simulink model "CCMSysServ"  (R2022b) (continued)
== system CCMSysServ == (continued)
      | BOSS SWITCH
      | <to>All</to>
      | <typ>Internal</typ>
      | <ReqID>NULL</ReqID>
      | <InitValue>0</InitValue>
  CarCfg_CarCfg47 : Inport  Description=|  Port=382  OutDataTypeStr=Nr2  PortDimensions=1
      | SELECTIVE CATALYTIC REDUCTION
      | <to>All</to>
      | <typ>Internal</typ>
      | <ReqID>NULL</ReqID>
      | <InitValue>0</InitValue>
  CarCfg_CarCfg470 : Inport  Description=|  Port=383  OutDataTypeStr=Nr2  PortDimensions=1
      | BELT PRE-TENSIONER 3RD ROW
      | <to>All</to>
      | <typ>Internal</typ>
      | <ReqID>NULL</ReqID>
      | <InitValue>0</InitValue>
  CarCfg_CarCfg471 : Inport  Description=|  Port=384  OutDataTypeStr=Nr2  PortDimensions=1
      | BACKREST MOTORS 1ST ROW
      | <to>All</to>
      | <typ>Internal</typ>
      | <ReqID>NULL</ReqID>
      | <InitValue>0</InitValue>
  CarCfg_CarCfg472 : Inport  Description=|  Port=385  OutDataTypeStr=Nr2  PortDimensions=1
      | FIRST ROW HEIGHT MOTORS
      | <to>All</to>
      | <typ>Internal</typ>
      | <ReqID>NULL</ReqID>
      | <InitValue>0</InitValue>
  CarCfg_CarCfg473 : Inport  Description=|  Port=386  OutDataTypeStr=Nr2  PortDimensions=1
      | SECOND ROW MASSAGE
      | <to>All</to>
      | <typ>Internal</typ>
      | <ReqID>NULL</ReqID>
      | <InitValue>0</InitValue>
  CarCfg_CarCfg474 : Inport  Description=|  Port=387  OutDataTypeStr=Nr2  PortDimensions=1
      | SECOND ROW POWER SEAT
      | <to>All</to>
      | <typ>Internal</typ>
      | <ReqID>NULL</ReqID>
      | <InitValue>0</InitValue>
  CarCfg_CarCfg475 : Inport  Description=|  Port=388  OutDataTypeStr=Nr2  PortDimensions=1
      | FIRST ROW LEG REST
      | <to>All</to>
      | <typ>Internal</typ>
      | <ReqID>NULL</ReqID>
      | <InitValue>0</InitValue>
  CarCfg_CarCfg476 : Inport  Description=|  Port=389  OutDataTypeStr=Nr2  PortDimensions=1
      | SECOND ROW LEG REST
      | <to>All</to>
      | <typ>Internal</typ>
      | <ReqID>NULL</ReqID>
      | <InitValue>0</InitValue>
  CarCfg_CarCfg477 : Inport  Description=|  Port=390  OutDataTypeStr=Nr2  PortDimensions=1
      | SECOND ROW EASY INGRESS/EGRESS
      | <to>All</to>
      | <typ>Internal</typ>
      | <ReqID>NULL</ReqID>
      | <InitValue>0</InitValue>
  CarCfg_CarCfg478 : Inport  Description=|  Port=391  OutDataTypeStr=Nr2  PortDimensions=1
      | DOOR OPENING WARNING
      | <to>All</to>
      | <typ>Internal</typ>
      | <ReqID>NULL</ReqID>
      | <InitValue>0</InitValue>
  CarCfg_CarCfg479 : Inport  Description=|  Port=392  OutDataTypeStr=Nr2  PortDimensions=1
      | FRONT CROSS TRAFFIC ALERT
      | <to>All</to>
      | <typ>Internal</typ>
      | <ReqID>NULL</ReqID>
      | <InitValue>0</InitValue>
  CarCfg_CarCfg48 : Inport  Description=|  Port=393  OutDataTypeStr=Nr2  PortDimensions=1
      | OIL LEVEL SENSOR
      | <to>All</to>
      | <typ>Internal</typ>
      | <ReqID>NULL</ReqID>
      | <InitValue>0</InitValue>
  CarCfg_CarCfg480 : Inport  Description=|  Port=394  OutDataTypeStr=Nr2  PortDimensions=1
      | NUMBER OF KEYLESS DOOR HANDLES
      | <to>All</to>
      | <typ>Internal</typ>
      | <ReqID>NULL</ReqID>
      | <InitValue>0</InitValue>
  CarCfg_CarCfg481 : Inport  Description=|  Port=395  OutDataTypeStr=Nr2  PortDimensions=1
      | POWER DOOR TYPE
      | <to>All</to>
      | <typ>Internal</typ>
      | <ReqID>NULL</ReqID>
      | <InitValue>0</InitValue>
  CarCfg_CarCfg482 : Inport  Description=|  Port=396  OutDataTypeStr=Nr2  PortDimensions=1
      | SEAT SPLIT SECOND ROW
      | <to>All</to>
      | <typ>Internal</typ>
      | <ReqID>NULL</ReqID>
      | <InitValue>0</InitValue>
  CarCfg_CarCfg483 : Inport  Description=|  Port=397  OutDataTypeStr=Nr2  PortDimensions=1
      | DIGITAL VIDEO RECORDER 
      | <to>All</to>
      | <typ>Internal</typ>
      | <ReqID>NULL</ReqID>
      | <InitValue>0</InitValue>
  CarCfg_CarCfg484 : Inport  Description=|  Port=398  OutDataTypeStr=Nr2  PortDimensions=1
      | LOGO LIGHT
      | <to>All</to>
      | <typ>Internal</typ>
      | <ReqID>NULL</ReqID>
      | <InitValue>0</InitValue>
  CarCfg_CarCfg485 : Inport  Description=|  Port=399  OutDataTypeStr=Nr2  PortDimensions=1
      | INTERIOR REAR VIEW MIRROR DISPLAY
      | <to>All</to>
      | <typ>Internal</typ>
      | <ReqID>NULL</ReqID>
      | <InitValue>0</InitValue>
  CarCfg_CarCfg486 : Inport  Description=|  Port=400  OutDataTypeStr=Nr2  PortDimensions=1
      | SECOND ROW LUMBAR AND SIDE SUPPORT
      | <to>All</to>
      | <typ>Internal</typ>
      | <ReqID>NULL</ReqID>
      | <InitValue>0</InitValue>
  CarCfg_CarCfg487 : Inport  Description=|  Port=401  OutDataTypeStr=Nr2  PortDimensions=1
      | BRAKE PEDAL ACTIVE DECOUPLING PYRO
      | <to>All</to>
      | <typ>Internal</typ>
      | <ReqID>NULL</ReqID>
      | <InitValue>0</InitValue>
  CarCfg_CarCfg488 : Inport  Description=|  Port=402  OutDataTypeStr=Nr2  PortDimensions=1
      | WIRELESS POWER TRANSFER MODULE
      | <to>All</to>
      | <typ>Internal</typ>
      | <ReqID>NULL</ReqID>
      | <InitValue>0</InitValue>
  CarCfg_CarCfg489 : Inport  Description=|  Port=403  OutDataTypeStr=Nr2  PortDimensions=1
      | BLE/NFC KEYS
      | <to>All</to>
      | <typ>Internal</typ>
      | <ReqID>NULL</ReqID>
      | <InitValue>0</InitValue>
  CarCfg_CarCfg49 : Inport  Description=|  Port=404  OutDataTypeStr=Nr2  PortDimensions=1
      | PERFORMANCE MODE
      | <to>All</to>
      | <typ>Internal</typ>
      | <ReqID>NULL</ReqID>
      | <InitValue>0</InitValue>
  CarCfg_CarCfg490 : Inport  Description=|  Port=405  OutDataTypeStr=Nr2  PortDimensions=1
      | OUTER OPENING TRIGGER OF TAILGATE
      | <to>All</to>
      | <typ>Internal</typ>
      | <ReqID>NULL</ReqID>
      | <InitValue>0</InitValue>
  CarCfg_CarCfg491 : Inport  Description=|  Port=406  OutDataTypeStr=Nr2  PortDimensions=1
      | YEAR EDITION
      | <to>All</to>
      | <typ>Internal</typ>
      | <ReqID>NULL</ReqID>
      | <InitValue>0</InitValue>
  CarCfg_CarCfg493 : Inport  Description=|  Port=407  OutDataTypeStr=Nr2  PortDimensions=1
      | EMERGENCY LANE OCCUPIED WARNING
      | <to>All</to>
      | <typ>Internal</typ>
      | <ReqID>NULL</ReqID>
      | <InitValue>0</InitValue>
  CarCfg_CarCfg494 : Inport  Description=|  Port=408  OutDataTypeStr=Nr2  PortDimensions=1
      | HIGHWAY ASSIST
      | <to>All</to>
      | <typ>Internal</typ>
      | <ReqID>NULL</ReqID>
      | <InitValue>0</InitValue>
  CarCfg_CarCfg495 : Inport  Description=|  Port=409  OutDataTypeStr=Nr2  PortDimensions=1
      | PARKING ASSISTANCE SUPPORT PEB
      | <to>All</to>
      | <typ>Internal</typ>
      | <ReqID>NULL</ReqID>
      | <InitValue>0</InitValue>
  CarCfg_CarCfg496 : Inport  Description=|  Port=410  OutDataTypeStr=Nr2  PortDimensions=1
      | BATTERY COOLANT LEVEL INDICATION
      | <to>All</to>
      | <typ>Internal</typ>
      | <ReqID>NULL</ReqID>
      | <InitValue>0</InitValue>
  CarCfg_CarCfg497 : Inport  Description=|  Port=411  OutDataTypeStr=Nr2  PortDimensions=1
      | INTERIOR VIEW CAMERA
      | <to>All</to>
      | <typ>Internal</typ>
      | <ReqID>NULL</ReqID>
      | <InitValue>0</InitValue>
  CarCfg_CarCfg498 : Inport  Description=|  Port=412  OutDataTypeStr=Nr2  PortDimensions=1
      | TEMPERATURE CONTROL CUP HOLDER
      | <to>All</to>
      | <typ>Internal</typ>
      | <ReqID>NULL</ReqID>
      | <InitValue>0</InitValue>
  CarCfg_CarCfg499 : Inport  Description=|  Port=413  OutDataTypeStr=Nr2  PortDimensions=1
      | INFOTAINMENT HEAD UNIT TYPE
      | <to>All</to>
      | <typ>Internal</typ>
      | <ReqID>NULL</ReqID>
      | <InitValue>0</InitValue>
  CarCfg_CarCfg5 : Inport  Description=|  Port=414  OutDataTypeStr=Nr2  PortDimensions=1
      | ENGINE
      | <to>All</to>
      | <typ>Internal</typ>
      | <ReqID>NULL</ReqID>
      | <InitValue>0</InitValue>
  CarCfg_CarCfg50 : Inport  Description=|  Port=415  OutDataTypeStr=Nr2  PortDimensions=1
      | CHASSIS TYPE
      | <to>All</to>
      | <typ>Internal</typ>
      | <ReqID>NULL</ReqID>
      | <InitValue>0</InitValue>
  CarCfg_CarCfg500 : Inport  Description=|  Port=416  OutDataTypeStr=Nr2  PortDimensions=1
      | DRIVER ALERT CONTROL
      | <to>All</to>
      | <typ>Internal</typ>
      | <ReqID>NULL</ReqID>
      | <InitValue>0</InitValue>
  CarCfg_CarCfg501 : Inport  Description=|  Port=417  OutDataTypeStr=Nr2  PortDimensions=1
      | WASHER FLUID LEVEL INDICATION FUNCTION
      | <to>All</to>
      | <typ>Internal</typ>
      | <ReqID>NULL</ReqID>
      | <InitValue>0</InitValue>
  CarCfg_CarCfg502 : Inport  Description=|  Port=418  OutDataTypeStr=Nr2  PortDimensions=1
      | ELECTRICAL PARK BRAKE HARD SWITCH
      | <to>All</to>
      | <typ>Internal</typ>
      | <ReqID>NULL</ReqID>
      | <InitValue>0</InitValue>
  CarCfg_CarCfg503 : Inport  Description=|  Port=419  OutDataTypeStr=Nr2  PortDimensions=1
      | WIPER MOTOR MODULE
      | <to>All</to>
      | <typ>Internal</typ>
      | <ReqID>NULL</ReqID>
      | <InitValue>0</InitValue>
  CarCfg_CarCfg504 : Inport  Description=|  Port=420  OutDataTypeStr=Nr2  PortDimensions=1
      | TRAFFIC LIGHT ATTENTION
      | <to>All</to>
      | <typ>Internal</typ>
      | <ReqID>NULL</ReqID>
      | <InitValue>0</InitValue>
  CarCfg_CarCfg505 : Inport  Description=|  Port=421  OutDataTypeStr=Nr2  PortDimensions=1
      | AIR FRAGRANCE
      | <to>All</to>
      | <typ>Internal</typ>
      | <ReqID>NULL</ReqID>
      | <InitValue>0</InitValue>
  CarCfg_CarCfg506 : Inport  Description=|  Port=422  OutDataTypeStr=Nr2  PortDimensions=1
      | ELECTRICAL VENTILATION ADJUSTMENT 
      | <to>All</to>
      | <typ>Internal</typ>
      | <ReqID>NULL</ReqID>
      | <InitValue>0</InitValue>
  CarCfg_CarCfg507 : Inport  Description=|  Port=423  OutDataTypeStr=Nr2  PortDimensions=1
      | HOOD TYPE
      | <to>All</to>
      | <typ>Internal</typ>
      | <ReqID>NULL</ReqID>
      | <InitValue>0</InitValue>
  CarCfg_CarCfg508 : Inport  Description=|  Port=424  OutDataTypeStr=Nr2  PortDimensions=1
      | REAR LAMP CONTROL MODULE
      | <to>All</to>
      | <typ>Internal</typ>
      | <ReqID>NULL</ReqID>
      | <InitValue>0</InitValue>
  CarCfg_CarCfg509 : Inport  Description=|  Port=425  OutDataTypeStr=Nr2  PortDimensions=1
      | HOME SAFE LIGHT
      | <to>All</to>
      | <typ>Internal</typ>
      | <ReqID>NULL</ReqID>
      | <InitValue>0</InitValue>
  CarCfg_CarCfg51 : Inport  Description=|  Port=426  OutDataTypeStr=Nr2  PortDimensions=1
      | TRAILER STABILITY ASSIST
      | <to>All</to>
      | <typ>Internal</typ>
      | <ReqID>NULL</ReqID>
      | <InitValue>0</InitValue>
  CarCfg_CarCfg510 : Inport  Description=|  Port=427  OutDataTypeStr=Nr2  PortDimensions=1
      | EXTERIOR LIGHT SHOW
      | <to>All</to>
      | <typ>Internal</typ>
      | <ReqID>NULL</ReqID>
      | <InitValue>0</InitValue>
  CarCfg_CarCfg511 : Inport  Description=|  Port=428  OutDataTypeStr=Nr2  PortDimensions=1
      | ADAPTIVE FRONT LIGHT SYSTEM
      | <to>All</to>
      | <typ>Internal</typ>
      | <ReqID>NULL</ReqID>
      | <InitValue>0</InitValue>
  CarCfg_CarCfg512 : Inport  Description=|  Port=429  OutDataTypeStr=Nr2  PortDimensions=1
      | SECOND BATTERY MONITORING SENSOR
      | <to>All</to>
      | <typ>Internal</typ>
      | <ReqID>NULL</ReqID>
      | <InitValue>0</InitValue>
  CarCfg_CarCfg513 : Inport  Description=|  Port=430  OutDataTypeStr=Nr2  PortDimensions=1
      | ENGINE COOLANT PUMP MODULE
      | <to>All</to>
      | <typ>Internal</typ>
      | <ReqID>NULL</ReqID>
      | <InitValue>0</InitValue>
  CarCfg_CarCfg514 : Inport  Description=|  Port=431  OutDataTypeStr=Nr2  PortDimensions=1
      | BATTERY COOLING PUMP FRONT MODULE
      | <to>All</to>
      | <typ>Internal</typ>
      | <ReqID>NULL</ReqID>
      | <InitValue>0</InitValue>
  CarCfg_CarCfg515 : Inport  Description=|  Port=432  OutDataTypeStr=Nr2  PortDimensions=1
      | HVAC BLOWER MODULE FRONT
      | <to>All</to>
      | <typ>Internal</typ>
      | <ReqID>NULL</ReqID>
      | <InitValue>0</InitValue>
  CarCfg_CarCfg516 : Inport  Description=|  Port=433  OutDataTypeStr=Nr2  PortDimensions=1
      | DRIVETRAIN COOLANT PUMP
      | <to>All</to>
      | <typ>Internal</typ>
      | <ReqID>NULL</ReqID>
      | <InitValue>0</InitValue>
  CarCfg_CarCfg517 : Inport  Description=|  Port=434  OutDataTypeStr=Nr2  PortDimensions=1
      | ELECTRIC DRIVETRAIN COOLANT PUMP
      | <to>All</to>
      | <typ>Internal</typ>
      | <ReqID>NULL</ReqID>
      | <InitValue>0</InitValue>
  CarCfg_CarCfg518 : Inport  Description=|  Port=435  OutDataTypeStr=Nr2  PortDimensions=1
      | OCCUPANT DETECTION SWITCHES 3RD ROW
      | <to>All</to>
      | <typ>Internal</typ>
      | <ReqID>NULL</ReqID>
      | <InitValue>0</InitValue>
  CarCfg_CarCfg519 : Inport  Description=|  Port=436  OutDataTypeStr=Nr2  PortDimensions=1
      | VEHICLE PERSONA THEME
      | <to>All</to>
      | <typ>Internal</typ>
      | <ReqID>NULL</ReqID>
      | <InitValue>0</InitValue>
  CarCfg_CarCfg52 : Inport  Description=|  Port=437  OutDataTypeStr=Nr2  PortDimensions=1
      | POST-IMPACT BRAKING
      | <to>All</to>
      | <typ>Internal</typ>
      | <ReqID>NULL</ReqID>
      | <InitValue>0</InitValue>
  CarCfg_CarCfg520 : Inport  Description=|  Port=438  OutDataTypeStr=Nr2  PortDimensions=1
      | 2ND ROW SIDE AIRBAGS
      | <to>All</to>
      | <typ>Internal</typ>
      | <ReqID>NULL</ReqID>
      | <InitValue>0</InitValue>
  CarCfg_CarCfg521 : Inport  Description=|  Port=439  OutDataTypeStr=Nr2  PortDimensions=1
      | SEAT MATERIAL FOR AUDIO TUNING
      | <to>All</to>
      | <typ>Internal</typ>
      | <ReqID>NULL</ReqID>
      | <InitValue>0</InitValue>
  CarCfg_CarCfg522 : Inport  Description=|  Port=440  OutDataTypeStr=Nr2  PortDimensions=1
      | 3RD ROW SEAT MATERIAL FOR AUDIO TUNING
      | <to>All</to>
      | <typ>Internal</typ>
      | <ReqID>NULL</ReqID>
      | <InitValue>0</InitValue>
  CarCfg_CarCfg523 : Inport  Description=|  Port=441  OutDataTypeStr=Nr2  PortDimensions=1
      | DRIVER DOOR SWITCH TYPE
      | <to>All</to>
      | <typ>Internal</typ>
      | <ReqID>NULL</ReqID>
      | <InitValue>0</InitValue>
  CarCfg_CarCfg524 : Inport  Description=|  Port=442  OutDataTypeStr=Nr2  PortDimensions=1
      | TRANSMISSION LOCKING
      | <to>All</to>
      | <typ>Internal</typ>
      | <ReqID>NULL</ReqID>
      | <InitValue>0</InitValue>
  CarCfg_CarCfg525 : Inport  Description=|  Port=443  OutDataTypeStr=Nr2  PortDimensions=1
      | MOOD LIGHT
      | <to>All</to>
      | <typ>Internal</typ>
      | <ReqID>NULL</ReqID>
      | <InitValue>0</InitValue>
  CarCfg_CarCfg526 : Inport  Description=|  Port=444  OutDataTypeStr=Nr2  PortDimensions=1
      | READING LIGHT
      | <to>All</to>
      | <typ>Internal</typ>
      | <ReqID>NULL</ReqID>
      | <InitValue>0</InitValue>
  CarCfg_CarCfg527 : Inport  Description=|  Port=445  OutDataTypeStr=Nr2  PortDimensions=1
      | VEHICLE TYPE
      | <to>All</to>
      | <typ>Internal</typ>
      | <ReqID>NULL</ReqID>
      | <InitValue>0</InitValue>
  CarCfg_CarCfg528 : Inport  Description=|  Port=446  OutDataTypeStr=Nr2  PortDimensions=1
      | VEHICLE TYPE
      | <to>All</to>
      | <typ>Internal</typ>
      | <ReqID>NULL</ReqID>
      | <InitValue>0</InitValue>
  CarCfg_CarCfg529 : Inport  Description=|  Port=447  OutDataTypeStr=Nr2  PortDimensions=1
      | VEHICLE TYPE
      | <to>All</to>
      | <typ>Internal</typ>
      | <ReqID>NULL</ReqID>
      | <InitValue>0</InitValue>
  CarCfg_CarCfg53 : Inport  Description=|  Port=448  OutDataTypeStr=Nr2  PortDimensions=1
      | HILL DESCENT CONTROL
      | <to>All</to>
      | <typ>Internal</typ>
      | <ReqID>NULL</ReqID>
      | <InitValue>0</InitValue>
  CarCfg_CarCfg531 : Inport  Description=|  Port=449  OutDataTypeStr=Nr2  PortDimensions=1
      | DRIVER DOOR AND LATCH TYPE
      | <to>All</to>
      | <typ>Internal</typ>
      | <ReqID>NULL</ReqID>
      | <InitValue>0</InitValue>
  CarCfg_CarCfg532 : Inport  Description=|  Port=450  OutDataTypeStr=Nr2  PortDimensions=1
      | PASSENGER DOOR AND LATCH TYPE
      | <to>All</to>
      | <typ>Internal</typ>
      | <ReqID>NULL</ReqID>
      | <InitValue>0</InitValue>
  CarCfg_CarCfg533 : Inport  Description=|  Port=451  OutDataTypeStr=Nr2  PortDimensions=1
      | REAR LEFT DOOR AND LATCH TYPE
      | <to>All</to>
      | <typ>Internal</typ>
      | <ReqID>NULL</ReqID>
      | <InitValue>0</InitValue>
  CarCfg_CarCfg534 : Inport  Description=|  Port=452  OutDataTypeStr=Nr2  PortDimensions=1
      | REAR RIGHT DOOR AND LATCH TYPE
      | <to>All</to>
      | <typ>Internal</typ>
      | <ReqID>NULL</ReqID>
      | <InitValue>0</InitValue>
  CarCfg_CarCfg535 : Inport  Description=|  Port=453  OutDataTypeStr=Nr2  PortDimensions=1
      | ELECTRICAL PARKING BRAKE CONTROL
      | <to>All</to>
      | <typ>Internal</typ>
      | <ReqID>947612</ReqID>
      | <InitValue>0</InitValue>
  CarCfg_CarCfg536 : Inport  Description=|  Port=454  OutDataTypeStr=Nr2  PortDimensions=1
      | AUTOSTART 2ND ROW CLIMATE
      | <to>All</to>
      | <typ>Internal</typ>
      | <ReqID>NULL</ReqID>
      | <InitValue>0</InitValue>
  CarCfg_CarCfg54 : Inport  Description=|  Port=455  OutDataTypeStr=Nr2  PortDimensions=1
      | WHEEL BRAKES TYPE REAR
      | <to>All</to>
      | <typ>Internal</typ>
      | <ReqID>NULL</ReqID>
      | <InitValue>0</InitValue>
  CarCfg_CarCfg540 : Inport  Port=456  OutDataTypeStr=Nr2  PortDimensions=1
  CarCfg_CarCfg541 : Inport  Description=|  Port=457  OutDataTypeStr=Nr2  PortDimensions=1
      | POW STEERING WHEEL POSITION ADJUSTMENT
      | <to>All</to>
      | <typ>Internal</typ>
      | <ReqID>NULL</ReqID>
      | <InitValue>0</InitValue>
  CarCfg_CarCfg543 : Inport  Description=|  Port=458  OutDataTypeStr=Nr2  PortDimensions=1
      | BATTERY BACKED-UP SOUNDER TYPE
      | <to>All</to>
      | <typ>Internal</typ>
      | <ReqID>NULL</ReqID>
      | <InitValue>0</InitValue>
  CarCfg_CarCfg544 : Inport  Description=|  Port=459  OutDataTypeStr=Nr2  PortDimensions=1
      | EXTERIOR REAR VIEW MIRROR DISPLAY
      | <to>All</to>
      | <typ>Internal</typ>
      | <ReqID>NULL</ReqID>
      | <InitValue>0</InitValue>
  CarCfg_CarCfg545 : Inport  Description=|  Port=460  OutDataTypeStr=Nr2  PortDimensions=1
      | BRAKE PEDAL SENSOR/SWITCH
      | <to>All</to>
      | <typ>Internal</typ>
      | <ReqID>NULL</ReqID>
      | <InitValue>0</InitValue>
  CarCfg_CarCfg546 : Inport  Description=|  Port=461  OutDataTypeStr=Nr2  PortDimensions=1
      | ILLUMINATION SWITCH TYPE
      | <to>All</to>
      | <typ>Internal</typ>
      | <ReqID>NULL</ReqID>
      | <InitValue>0</InitValue>
  CarCfg_CarCfg547 : Inport  Port=462  OutDataTypeStr=Nr2  PortDimensions=1
  CarCfg_CarCfg55 : Inport  Description=|  Port=463  OutDataTypeStr=Nr2  PortDimensions=1
      | WHEEL BRAKES TYPE FRONT
      | <to>All</to>
      | <typ>Internal</typ>
      | <ReqID>NULL</ReqID>
      | <InitValue>0</InitValue>
  CarCfg_CarCfg550 : Inport  Description=|  Port=464  OutDataTypeStr=Nr2  PortDimensions=1
      | LUMBAR CONTROL
      | <to>All</to>
      | <typ>Internal</typ>
      | <ReqID>667104</ReqID>
      | <InitValue>0</InitValue>
  CarCfg_CarCfg551 : Inport  Description=|  Port=465  OutDataTypeStr=Nr2  PortDimensions=1
      | SECOND ROW MEMORY SEAT
      | <to>All</to>
      | <typ>Internal</typ>
      | <ReqID>NULL</ReqID>
      | <InitValue>0</InitValue>
  CarCfg_CarCfg56 : Inport  Description=|  Port=466  OutDataTypeStr=Nr2  PortDimensions=1
      | BRAKE PADS
      | <to>All</to>
      | <typ>Internal</typ>
      | <ReqID>NULL</ReqID>
      | <InitValue>0</InitValue>
  CarCfg_CarCfg561 : Inport  Description=|  Port=467  OutDataTypeStr=Nr2  PortDimensions=1
      | DOOR WINDOWS TYPE
      | <to>All</to>
      | <typ>Internal</typ>
      | <ReqID>NULL</ReqID>
      | <InitValue>0</InitValue>
  CarCfg_CarCfg564 : Inport  Description=|  Port=468  OutDataTypeStr=Nr2  PortDimensions=1
      | ACTIVE REAR SPOILER
      | <to>All</to>
      | <typ>Internal</typ>
      | <ReqID>NULL</ReqID>
      | <InitValue>0</InitValue>
  CarCfg_CarCfg565 : Inport  Description=|  Port=469  OutDataTypeStr=Nr2  PortDimensions=1
      | REMOTE STRAIGHT PARKING ASSIST
      | <to>All</to>
      | <typ>Internal</typ>
      | <ReqID>NULL</ReqID>
      | <InitValue>0</InitValue>
  CarCfg_CarCfg566 : Inport  Description=|  Port=470  OutDataTypeStr=Nr2  PortDimensions=1
      | VEHICLE TYPE
      | <to>All</to>
      | <typ>Internal</typ>
      | <ReqID>NULL</ReqID>
      | <InitValue>0</InitValue>
  CarCfg_CarCfg567 : Inport  Description=|  Port=471  OutDataTypeStr=Nr2  PortDimensions=1
      | ELECTRIC VEHICLE INLET TYPE
      | <to>All</to>
      | <typ>Internal</typ>
      | <ReqID>NULL</ReqID>
      | <InitValue>0</InitValue>
  CarCfg_CarCfg568 : Inport  Description=|  Port=472  OutDataTypeStr=Nr2  PortDimensions=1
      | LIFE DETECTION AND CARE
      | <to>All</to>
      | <typ>Internal</typ>
      | <ReqID>NULL</ReqID>
      | <InitValue>0</InitValue>
  CarCfg_CarCfg569 : Inport  Description=|  Port=473  OutDataTypeStr=Nr2  PortDimensions=1
      | REDUNDANT LV ENERGY SOURCE
      | <to>All</to>
      | <typ>Internal</typ>
      | <ReqID>21966</ReqID>
      | <InitValue>0</InitValue>
  CarCfg_CarCfg57 : Inport  Description=|  Port=474  OutDataTypeStr=Nr2  PortDimensions=1
      | DRIVE MODE
      | <to>All</to>
      | <typ>Internal</typ>
      | <ReqID>NULL</ReqID>
      | <InitValue>0</InitValue>
  CarCfg_CarCfg578 : Inport  Description=|  Port=475  OutDataTypeStr=Nr2  PortDimensions=1
      | DC CHARGE LID LOCK
      | <to>All</to>
      | <typ>Internal</typ>
      | <ReqID>NULL</ReqID>
      | <InitValue>0</InitValue>
  CarCfg_CarCfg579 : Inport  Description=|  Port=476  OutDataTypeStr=Nr2  PortDimensions=1
      | VEHICLE TYPE
      | <to>All</to>
      | <typ>Internal</typ>
      | <ReqID>NULL</ReqID>
      | <InitValue>0</InitValue>
  CarCfg_CarCfg58 : Inport  Description=|  Port=477  OutDataTypeStr=Nr2  PortDimensions=1
      | AIR SUSPENSION
      | <to>All</to>
      | <typ>Internal</typ>
      | <ReqID>NULL</ReqID>
      | <InitValue>0</InitValue>
  CarCfg_CarCfg580 : Inport  Description=|  Port=478  OutDataTypeStr=Nr2  PortDimensions=1
      | VEHICLE TYPE
      | <to>All</to>
      | <typ>Internal</typ>
      | <ReqID>NULL</ReqID>
      | <InitValue>0</InitValue>
  CarCfg_CarCfg59 : Inport  Description=|  Port=479  OutDataTypeStr=Nr2  PortDimensions=1
      | ACTIVE SUSPENSION
      | <to>All</to>
      | <typ>Internal</typ>
      | <ReqID>NULL</ReqID>
      | <InitValue>0</InitValue>
  CarCfg_CarCfg590 : Inport  Description=|  Port=480  OutDataTypeStr=Nr2  PortDimensions=1
      | MAIN FUEL OR CHARGE LID LOCATION
      | <to>All</to>
      | <typ>Internal</typ>
      | <ReqID>56442</ReqID>
      | <InitValue>0</InitValue>
  CarCfg_CarCfg591 : Inport  Description=|  Port=481  OutDataTypeStr=Nr2  PortDimensions=1
      | SECOND CHARGE LID LOCATION
      | <to>All</to>
      | <typ>Internal</typ>
      | <ReqID>56442</ReqID>
      | <InitValue>0</InitValue>
  CarCfg_CarCfg593 : Inport  Description=|  Port=482  OutDataTypeStr=Nr2  PortDimensions=1
      | SUPPORT LV ENERGY SOURCE
      | <to>All</to>
      | <typ>Internal</typ>
      | <ReqID>NULL</ReqID>
      | <InitValue>0</InitValue>
  CarCfg_CarCfg595 : Inport  Description=|  Port=483  OutDataTypeStr=Nr2  PortDimensions=1
      | Architecture type of all-electric charging cover
      | <to>All</to>
      | <typ>Internal</typ>
      | <ReqID>NULL</ReqID>
      | <InitValue>0</InitValue>
  CarCfg_CarCfg599 : Inport  Description=|  Port=484  OutDataTypeStr=Nr2  PortDimensions=1
      | VEHICLE TYPE
      | <to>All</to>
      | <typ>Internal</typ>
      | <ReqID>NULL</ReqID>
      | <InitValue>0</InitValue>
  CarCfg_CarCfg6 : Inport  Description=|  Port=485  OutDataTypeStr=Nr2  PortDimensions=1
      | FUEL
      | <to>All</to>
      | <typ>Internal</typ>
      | <ReqID>NULL</ReqID>
      | <InitValue>0</InitValue>
  CarCfg_CarCfg60 : Inport  Description=|  Port=486  OutDataTypeStr=Nr2  PortDimensions=1
      | LEVELLING SENSOR CONFIGURATION
      | <to>All</to>
      | <typ>Internal</typ>
      | <ReqID>NULL</ReqID>
      | <InitValue>0</InitValue>
  CarCfg_CarCfg604 : Inport  Description=|  Port=487  OutDataTypeStr=Nr2  PortDimensions=1
      | VEHICLE TYPE
      | <to>All</to>
      | <typ>Internal</typ>
      | <ReqID>NULL</ReqID>
      | <InitValue>0</InitValue>
  CarCfg_CarCfg609 : Inport  Description=|  Port=488  OutDataTypeStr=Nr2  PortDimensions=1
      | VEHICLE TYPE
      | <to>All</to>
      | <typ>Internal</typ>
      | <ReqID>NULL</ReqID>
      | <InitValue>0</InitValue>
  CarCfg_CarCfg61 : Inport  Description=|  Port=489  OutDataTypeStr=Nr2  PortDimensions=1
      | HILL START ASSIST
      | <to>All</to>
      | <typ>Internal</typ>
      | <ReqID>NULL</ReqID>
      | <InitValue>0</InitValue>
  CarCfg_CarCfg611 : Inport  Description=|  Port=490  OutDataTypeStr=Nr2  PortDimensions=1
      | Refresh mode
      | <to>All</to>
      | <typ>Internal</typ>
      | <ReqID>NULL</ReqID>
      | <InitValue>0</InitValue>
  CarCfg_CarCfg613 : Inport  Description=|  Port=491  OutDataTypeStr=Nr2  PortDimensions=1
      | Romantic Mode
      | <to>All</to>
      | <typ>Internal</typ>
      | <ReqID>NULL</ReqID>
      | <InitValue>0</InitValue>
  CarCfg_CarCfg615 : Inport  Description=|  Port=492  OutDataTypeStr=Nr2  PortDimensions=1
      | ECO+ mode
      | <to>All</to>
      | <typ>Internal</typ>
      | <ReqID>NULL</ReqID>
      | <InitValue>0</InitValue>
  CarCfg_CarCfg616 : Inport  Description=|  Port=493  OutDataTypeStr=Nr2  PortDimensions=1
      | Rest Mode
      | <to>All</to>
      | <typ>Internal</typ>
      | <ReqID>NULL</ReqID>
      | <InitValue>0</InitValue>
  CarCfg_CarCfg617 : Inport  Description=|  Port=494  OutDataTypeStr=Nr2  PortDimensions=1
      | Theater mode
      | <to>All</to>
      | <typ>Internal</typ>
      | <ReqID>NULL</ReqID>
      | <InitValue>0</InitValue>
  CarCfg_CarCfg618 : Inport  Description=|  Port=495  OutDataTypeStr=Nr2  PortDimensions=1
      | Vehicle Sentry and Theft Deterrence
      | <to>UsgModMgr</to>
      | <typ>Internal</typ>
      | <ReqID>22019</ReqID>
      | <InitValue>0</InitValue>
  CarCfg_CarCfg62 : Inport  Description=|  Port=496  OutDataTypeStr=Nr2  PortDimensions=1
      | FRONT AXLE WEIGHT
      | <to>All</to>
      | <typ>Internal</typ>
      | <ReqID>NULL</ReqID>
      | <InitValue>0</InitValue>
  CarCfg_CarCfg622 : Inport  Description=|  Port=497  OutDataTypeStr=Nr2  PortDimensions=1
      | Grille Lamp
      | <to>All</to>
      | <typ>Internal</typ>
      | <ReqID>682377</ReqID>
      | <InitValue>0</InitValue>
  CarCfg_CarCfg624 : Inport  Description=|  Port=498  OutDataTypeStr=Nr2  PortDimensions=1
      | RPA TYPE
      | <to>All</to>
      | <typ>Internal</typ>
      | <ReqID>NULL</ReqID>
      | <InitValue>0</InitValue>
  CarCfg_CarCfg626 : Inport  Description=|  Port=499  OutDataTypeStr=Nr2  PortDimensions=1
      | Customization mode
      | <to>All</to>
      | <typ>Internal</typ>
      | <ReqID>NULL</ReqID>
      | <InitValue>0</InitValue>
  CarCfg_CarCfg627 : Inport  Description=|  Port=500  OutDataTypeStr=Nr2  PortDimensions=1
      | Meeting Mode
      | <to>All</to>
      | <typ>Internal</typ>
      | <ReqID>NULL</ReqID>
      | <InitValue>0</InitValue>
  CarCfg_CarCfg628 : Inport  Description=|  Port=501  OutDataTypeStr=Nr2  PortDimensions=1
      | Digital Key Protocol
      | <to>All</to>
      | <typ>Internal</typ>
      | <ReqID>NULL</ReqID>
      | <InitValue>0</InitValue>
  CarCfg_CarCfg629 : Inport  Description=|  Port=502  OutDataTypeStr=Nr2  PortDimensions=1
      | EXTERIOR Light Switch Type
      | <to>All</to>
      | <typ>Internal</typ>
      | <ReqID>21928</ReqID>
      | <InitValue>0</InitValue>
  CarCfg_CarCfg63 : Inport  Description=|  Port=503  OutDataTypeStr=Nr2  PortDimensions=1
      | REAR AXLE WEIGHT
      | <to>All</to>
      | <typ>Internal</typ>
      | <ReqID>NULL</ReqID>
      | <InitValue>0</InitValue>
  CarCfg_CarCfg630 : Inport  Description=|  Port=504  OutDataTypeStr=Nr2  PortDimensions=1
      | Remote Pilot Function
      | <to>All</to>
      | <typ>Internal</typ>
      | <ReqID>267298</ReqID>
      | <InitValue>0</InitValue>
  CarCfg_CarCfg634 : Inport  Description=|  Port=505  OutDataTypeStr=Nr2  PortDimensions=1
      | RPA TYPE
      | <to>All</to>
      | <typ>Internal</typ>
      | <ReqID>NULL</ReqID>
      | <InitValue>0</InitValue>
  CarCfg_CarCfg635 : Inport  Description=|  Port=506  OutDataTypeStr=Nr2  PortDimensions=1
      | Architecture type of all-electric charging cover
      | <to>All</to>
      | <typ>Internal</typ>
      | <ReqID>NULL</ReqID>
      | <InitValue>0</InitValue>
  CarCfg_CarCfg639 : Inport  Description=|  Port=507  OutDataTypeStr=Nr2  PortDimensions=1
      | AVP TYPE
      | <to>All</to>
      | <typ>Internal</typ>
      | <ReqID>NULL</ReqID>
      | <InitValue>0</InitValue>
  CarCfg_CarCfg64 : Inport  Description=|  Port=508  OutDataTypeStr=Nr2  PortDimensions=1
      | ALARM
      | <to>All</to>
      | <typ>Internal</typ>
      | <ReqID>NULL</ReqID>
      | <InitValue>0</InitValue>
  CarCfg_CarCfg640 : Inport  Description=|  Port=509  OutDataTypeStr=Nr2  PortDimensions=1
      | RPA TYPE
      | <to>All</to>
      | <typ>Internal</typ>
      | <ReqID>NULL</ReqID>
      | <InitValue>0</InitValue>
  CarCfg_CarCfg642 : Inport  Description=|  Port=510  OutDataTypeStr=Nr2  PortDimensions=1
      | Active rear spoiler type
      | <to>All</to>
      | <typ>Internal</typ>
      | <ReqID>512424</ReqID>
      | <InitValue>0</InitValue>
  CarCfg_CarCfg644 : Inport  Description=|  Port=511  OutDataTypeStr=Nr2  PortDimensions=1
      | Architecture type of all-electric charging cover
      | <to>All</to>
      | <typ>Internal</typ>
      | <ReqID>NULL</ReqID>
      | <InitValue>0</InitValue>
  CarCfg_CarCfg645 : Inport  Description=|  Port=512  OutDataTypeStr=Nr2  PortDimensions=1
      | Architecture type of all-electric charging cover
      | <to>All</to>
      | <typ>Internal</typ>
      | <ReqID>NULL</ReqID>
      | <InitValue>0</InitValue>
  CarCfg_CarCfg646 : Inport  Description=|  Port=513  OutDataTypeStr=Nr2  PortDimensions=1
      | Architecture type of all-electric charging cover
      | <to>All</to>
      | <typ>Internal</typ>
      | <ReqID>NULL</ReqID>
      | <InitValue>0</InitValue>
  CarCfg_CarCfg647 : Inport  Description=|  Port=514  OutDataTypeStr=Nr2  PortDimensions=1
      | Side step light
      | <to>All</to>
      | <typ>Internal</typ>
      | <ReqID>56442</ReqID>
      | <InitValue>0</InitValue>
  CarCfg_CarCfg648 : Inport  Description=|  Port=515  OutDataTypeStr=Nr2  PortDimensions=1
      | VEHICLE TYPE
      | <to>All</to>
      | <typ>Internal</typ>
      | <ReqID>NULL</ReqID>
      | <InitValue>0</InitValue>
  CarCfg_CarCfg65 : Inport  Description=|  Port=516  OutDataTypeStr=Nr2  PortDimensions=1
      | INTERIOR MOTION SENSOR
      | <to>All</to>
      | <typ>Internal</typ>
      | <ReqID>NULL</ReqID>
      | <InitValue>0</InitValue>
  CarCfg_CarCfg650 : Inport  Description=|  Port=517  OutDataTypeStr=Nr2  PortDimensions=1
      | EXTERNAL VOICE INTERACTION
      | <to>All</to>
      | <typ>Internal</typ>
      | <ReqID>NULL</ReqID>
      | <InitValue>0</InitValue>
  CarCfg_CarCfg651 : Inport  Description=|  Port=518  OutDataTypeStr=Nr2  PortDimensions=1
      | ELECTRIC VEHICLE INLET TYPE
      | <to>All</to>
      | <typ>Internal</typ>
      | <ReqID>NULL</ReqID>
      | <InitValue>0</InitValue>
  CarCfg_CarCfg652 : Inport  Description=|  Port=519  OutDataTypeStr=Nr2  PortDimensions=1
      | Rear door handle switch type
      | <to>All</to>
      | <typ>Internal</typ>
      | <ReqID>NULL</ReqID>
      | <InitValue>0</InitValue>
  CarCfg_CarCfg659 : Inport  Description=|  Port=520  OutDataTypeStr=Nr2  PortDimensions=1
      | VEHICLE TYPE
      | <to>All</to>
      | <typ>Internal</typ>
      | <ReqID>NULL</ReqID>
      | <InitValue>0</InitValue>
  CarCfg_CarCfg66 : Inport  Description=|  Port=521  OutDataTypeStr=Nr2  PortDimensions=1
      | INCLINATION SENSOR
      | <to>All</to>
      | <typ>Internal</typ>
      | <ReqID>NULL</ReqID>
      | <InitValue>0</InitValue>
  CarCfg_CarCfg660 : Inport  Description=|  Port=522  OutDataTypeStr=Nr2  PortDimensions=1
      | VEHICLE TYPE
      | <to>All</to>
      | <typ>Internal</typ>
      | <ReqID>NULL</ReqID>
      | <InitValue>0</InitValue>
  CarCfg_CarCfg665 : Inport  Description=|  Port=523  OutDataTypeStr=Nr2  PortDimensions=1
      | VEHICLE TYPE
      | <to>All</to>
      | <typ>Internal</typ>
      | <ReqID>NULL</ReqID>
      | <InitValue>0</InitValue>
  CarCfg_CarCfg67 : Inport  Description=|  Port=524  OutDataTypeStr=Nr2  PortDimensions=1
      | STEERING COLUMN LOCK
      | <to>All</to>
      | <typ>Internal</typ>
      | <ReqID>NULL</ReqID>
      | <InitValue>0</InitValue>
  CarCfg_CarCfg671 : Inport  Description=|  Port=525  OutDataTypeStr=Nr2  PortDimensions=1
      | Generation of power side door
      | <to>All</to>
      | <typ>Internal</typ>
      | <ReqID>301295</ReqID>
      | <InitValue>0</InitValue>
  CarCfg_CarCfg672 : Inport  Description=|  Port=526  OutDataTypeStr=Nr2  PortDimensions=1
      | Gesture Interaction
      | <to>All</to>
      | <typ>Internal</typ>
      | <ReqID>526878</ReqID>
      | <InitValue>0</InitValue>
  CarCfg_CarCfg675 : Inport  Description=|  Port=527  OutDataTypeStr=Nr2  PortDimensions=1
      | ELECTRIC RETRACTABLE STEP
      | <to>All</to>
      | <typ>Internal</typ>
      | <ReqID>56442</ReqID>
      | <InitValue>0</InitValue>
  CarCfg_CarCfg677 : Inport  Port=528  OutDataTypeStr=Nr2  PortDimensions=1
  CarCfg_CarCfg678 : Inport  Port=529  OutDataTypeStr=Nr2  PortDimensions=1
  CarCfg_CarCfg68 : Inport  Description=|  Port=530  OutDataTypeStr=Nr2  PortDimensions=1
      | PANIC FUNCTION
      | <to>All</to>
      | <typ>Internal</typ>
      | <ReqID>NULL</ReqID>
      | <InitValue>0</InitValue>
  CarCfg_CarCfg683 : Inport  Description=|  Port=531  OutDataTypeStr=Nr2  PortDimensions=1
      | /
      | <to>All</to>
      | <typ>Internal</typ>
      | <ReqID>NULL</ReqID>
      | <InitValue>0</InitValue>
  CarCfg_CarCfg684 : Inport  Description=|  Port=532  OutDataTypeStr=Nr2  PortDimensions=1
      | VEHICLE TYPE
      | <to>All</to>
      | <typ>Internal</typ>
      | <ReqID>NULL</ReqID>
      | <InitValue>0</InitValue>
  CarCfg_CarCfg686 : Inport  Description=|  Port=533  OutDataTypeStr=Nr2  PortDimensions=1
      | Architecture type of all-electric charging cover
      | <to>All</to>
      | <typ>Internal</typ>
      | <ReqID>NULL</ReqID>
      | <InitValue>0</InitValue>
  CarCfg_CarCfg687 : Inport  Description=|  Port=534  OutDataTypeStr=Nr2  PortDimensions=1
      | Architecture type of all-electric charging cover
      | <to>All</to>
      | <typ>Internal</typ>
      | <ReqID>NULL</ReqID>
      | <InitValue>0</InitValue>
  CarCfg_CarCfg688 : Inport  Description=|  Port=535  OutDataTypeStr=Nr2  PortDimensions=1
      | VEHICLE TYPE
      | <to>All</to>
      | <typ>Internal</typ>
      | <ReqID>NULL</ReqID>
      | <InitValue>0</InitValue>
  CarCfg_CarCfg69 : Inport  Description=|  Port=536  OutDataTypeStr=Nr2  PortDimensions=1
      | RETRIGG ENABLING, IF ALARM
      | <to>All</to>
      | <typ>Internal</typ>
      | <ReqID>NULL</ReqID>
      | <InitValue>0</InitValue>
  CarCfg_CarCfg695 : Inport  Description=|  Port=537  OutDataTypeStr=Nr2  PortDimensions=1
      | VEHICLE TYPE
      | <to>All</to>
      | <typ>Internal</typ>
      | <ReqID>NULL</ReqID>
      | <InitValue>0</InitValue>
  CarCfg_CarCfg697 : Inport  Description=|  Port=538  OutDataTypeStr=Nr2  PortDimensions=1
      | VEHICLE TYPE
      | <to>All</to>
      | <typ>Internal</typ>
      | <ReqID>NULL</ReqID>
      | <InitValue>0</InitValue>
  CarCfg_CarCfg698 : Inport  Description=|  Port=539  OutDataTypeStr=Nr2  PortDimensions=1
      | Architecture type of all-electric charging cover
      | <to>All</to>
      | <typ>Internal</typ>
      | <ReqID>NULL</ReqID>
      | <InitValue>0</InitValue>
  CarCfg_CarCfg699 : Inport  Description=|  Port=540  OutDataTypeStr=Nr2  PortDimensions=1
      | Wading Assist System
      | <to>All</to>
      | <typ>Internal</typ>
      | <ReqID>710182</ReqID>
      | <InitValue>0</InitValue>
  CarCfg_CarCfg70 : Inport  Description=|  Port=541  OutDataTypeStr=Nr2  PortDimensions=1
      | PASSIVE ARMING
      | <to>All</to>
      | <typ>Internal</typ>
      | <ReqID>NULL</ReqID>
      | <InitValue>0</InitValue>
  CarCfg_CarCfg701 : Inport  Description=|  Port=542  OutDataTypeStr=Nr2  PortDimensions=1
      | VEHICLE TYPE
      | <to>All</to>
      | <typ>Internal</typ>
      | <ReqID>NULL</ReqID>
      | <InitValue>0</InitValue>
  CarCfg_CarCfg705 : Inport  Description=|  Port=543  OutDataTypeStr=Nr2  PortDimensions=1
      | Architecture type of all-electric charging cover
      | <to>All</to>
      | <typ>Internal</typ>
      | <ReqID>NULL</ReqID>
      | <InitValue>0</InitValue>
  CarCfg_CarCfg709 : Inport  Description=|  Port=544  OutDataTypeStr=Nr2  PortDimensions=1
      | BRAND
      | <to>All</to>
      | <typ>Internal</typ>
      | <ReqID>700858</ReqID>
      | <InitValue>0</InitValue>
  CarCfg_CarCfg71 : Inport  Description=|  Port=545  OutDataTypeStr=Nr2  PortDimensions=1
      | FOREIGN COMPONENT DETECTION
      | <to>All</to>
      | <typ>Internal</typ>
      | <ReqID>NULL</ReqID>
      | <InitValue>0</InitValue>
  CarCfg_CarCfg714 : Inport  Description=|  Port=546  OutDataTypeStr=Nr2  PortDimensions=1
      | Rear Windshild electric Heating Defrosting
      | <to>All</to>
      | <typ>Internal</typ>
      | <ReqID>86032</ReqID>
      | <InitValue>0</InitValue>
  CarCfg_CarCfg715 : Inport  Description=|  Port=547  OutDataTypeStr=Nr2  PortDimensions=1
      | Lighting scene mode
      | <to>All</to>
      | <typ>Internal</typ>
      | <ReqID>699482</ReqID>
      | <InitValue>0</InitValue>
  CarCfg_CarCfg718 : Inport  Description=|  Port=548  OutDataTypeStr=Nr2  PortDimensions=1
      | /
      | <to>All</to>
      | <typ>Internal</typ>
      | <ReqID>NULL</ReqID>
      | <InitValue>0</InitValue>
  CarCfg_CarCfg719 : Inport  Description=|  Port=549  OutDataTypeStr=Nr2  PortDimensions=1
      | Architecture type of all-electric charging cover
      | <to>All</to>
      | <typ>Internal</typ>
      | <ReqID>NULL</ReqID>
      | <InitValue>0</InitValue>
  CarCfg_CarCfg72 : Inport  Description=|  Port=550  OutDataTypeStr=Nr2  PortDimensions=1
      | IMMOBILISER VARIANT
      | <to>All</to>
      | <typ>Internal</typ>
      | <ReqID>NULL</ReqID>
      | <InitValue>0</InitValue>
  CarCfg_CarCfg720 : Inport  Description=|  Port=551  OutDataTypeStr=Nr2  PortDimensions=1
      | TEMPERED STORAGE BOX
      | <to>ThmCtrl</to>
      | <typ>Internal</typ>
      | <ReqID>987440987442</ReqID>
      | <InitValue>0</InitValue>
  CarCfg_CarCfg721 : Inport  Description=|  Port=552  OutDataTypeStr=Nr2  PortDimensions=1
      | VEHICLE TYPE
      | <to>All</to>
      | <typ>Internal</typ>
      | <ReqID>NULL</ReqID>
      | <InitValue>0</InitValue>
  CarCfg_CarCfg726 : Inport  Description=|  Port=553  OutDataTypeStr=Nr2  PortDimensions=1
      | EASY INGRESS EGRESS FOR PASSENGER   
      | <to>All</to>
      | <typ>Internal</typ>
      | <ReqID>NULL</ReqID>
      | <InitValue>0</InitValue>
  CarCfg_CarCfg73 : Inport  Description=|  Port=554  OutDataTypeStr=Nr2  PortDimensions=1
      | KEY WARNING
      | <to>All</to>
      | <typ>Internal</typ>
      | <ReqID>NULL</ReqID>
      | <InitValue>0</InitValue>
  CarCfg_CarCfg732 : Inport  Description=|  Port=555  OutDataTypeStr=Nr2  PortDimensions=1
      | VEHICLE TYPE
      | <to>All</to>
      | <typ>Internal</typ>
      | <ReqID>NULL</ReqID>
      | <InitValue>0</InitValue>
  CarCfg_CarCfg733 : Inport  Description=|  Port=556  OutDataTypeStr=Nr2  PortDimensions=1
      | STEERING BY WIRE TYPE
      | <to>DiSysCtl</to>
      | <typ>Internal</typ>
      | <ReqID>225609</ReqID>
      | <InitValue>0</InitValue>
  CarCfg_CarCfg734 : Inport  Description=|  Port=557  OutDataTypeStr=Nr2  PortDimensions=1
      | VEHICLE TYPE
      | <to>All</to>
      | <typ>Internal</typ>
      | <ReqID>NULL</ReqID>
      | <InitValue>0</InitValue>
  CarCfg_CarCfg74 : Inport  Description=|  Port=558  OutDataTypeStr=Nr2  PortDimensions=1
      | ATTENUATION UPHOLSTERY
      | <to>All</to>
      | <typ>Internal</typ>
      | <ReqID>NULL</ReqID>
      | <InitValue>0</InitValue>
  CarCfg_CarCfg740 : Inport  Port=559  OutDataTypeStr=Nr2  PortDimensions=1
  CarCfg_CarCfg741 : Inport  Description=|  Port=560  OutDataTypeStr=Nr2  PortDimensions=1
      | Rear Window Defroster Control General Electrical Failures Circuit short to battery
      | <to>All</to>
      | <typ>Internal</typ>
      | <ReqID>947612</ReqID>
      | <InitValue>0</InitValue>
  CarCfg_CarCfg742 : Inport  Description=|  Port=561  OutDataTypeStr=Nr2  PortDimensions=1
      | VEHICLE TYPE
      | <to>All</to>
      | <typ>Internal</typ>
      | <ReqID>NULL</ReqID>
      | <InitValue>0</InitValue>
  CarCfg_CarCfg746 : Inport  Description=|  Port=562  OutDataTypeStr=Nr2  PortDimensions=1
      | MARKET
      | <to>All</to>
      | <typ>Internal</typ>
      | <ReqID>56442</ReqID>
      | <InitValue>0</InitValue>
  CarCfg_CarCfg75 : Inport  Description=|  Port=563  OutDataTypeStr=Nr2  PortDimensions=1
      | THEFT NOTIFICATION
      | <to>All</to>
      | <typ>Internal</typ>
      | <ReqID>NULL</ReqID>
      | <InitValue>0</InitValue>
  CarCfg_CarCfg753 : Inport  Description=|  Port=564  OutDataTypeStr=Nr2  PortDimensions=1
      | Side Logo Light
      | <to>All</to>
      | <typ>Internal</typ>
      | <ReqID>677462</ReqID>
      | <InitValue>0</InitValue>
  CarCfg_CarCfg755 : Inport  Description=|  Port=565  OutDataTypeStr=Nr2  PortDimensions=1
      | Number of rear turn lamp
      | <to>All</to>
      | <typ>Internal</typ>
      | <ReqID>677230</ReqID>
      | <InitValue>0</InitValue>
  CarCfg_CarCfg756 : Inport  Description=|  Port=566  OutDataTypeStr=Nr2  PortDimensions=1
      | Auto Drive Marker Lamp
      | <to>All</to>
      | <typ>Internal</typ>
      | <ReqID>/</ReqID>
      | <InitValue>0</InitValue>
  CarCfg_CarCfg76 : Inport  Description=|  Port=567  OutDataTypeStr=Nr2  PortDimensions=1
      | STOLEN VEHICLE TRACKING
      | <to>All</to>
      | <typ>Internal</typ>
      | <ReqID>NULL</ReqID>
      | <InitValue>0</InitValue>
  CarCfg_CarCfg763 : Inport  Description=|  Port=568  OutDataTypeStr=Nr2  PortDimensions=1
      | CONTAINER COVER
      | <to>All</to>
      | <typ>Internal</typ>
      | <ReqID>198565</ReqID>
      | <InitValue>0</InitValue>
  CarCfg_CarCfg766 : Inport  Description=|  Port=569  OutDataTypeStr=Nr2  PortDimensions=1
      | EMERGENCY DOOR OPENING
      | <to>All</to>
      | <typ>Internal</typ>
      | <ReqID>198565</ReqID>
      | <InitValue>0</InitValue>
  CarCfg_CarCfg768 : Inport  Description=|  Port=570  OutDataTypeStr=Nr2  PortDimensions=1
      | License plate light rear
      | <to>All</to>
      | <typ>Internal</typ>
      | <ReqID>174900</ReqID>
      | <InitValue>0</InitValue>
  CarCfg_CarCfg77 : Inport  Description=|  Port=571  OutDataTypeStr=Nr2  PortDimensions=1
      | ASSISTANCE SERVICES
      | <to>All</to>
      | <typ>Internal</typ>
      | <ReqID>NULL</ReqID>
      | <InitValue>0</InitValue>
  CarCfg_CarCfg770 : Inport  Description=|  Port=572  OutDataTypeStr=Nr2  PortDimensions=1
      | CATEGORY OF VEHICLE
      | <to>All</to>
      | <typ>Internal</typ>
      | <ReqID>174900</ReqID>
      | <InitValue>0</InitValue>
  CarCfg_CarCfg773 : Inport  Description=|  Port=573  OutDataTypeStr=Nr2  PortDimensions=1
      | Remote Control Vehicle
      | <to>All</to>
      | <typ>Internal</typ>
      | <ReqID>700858</ReqID>
      | <InitValue>0</InitValue>
  CarCfg_CarCfg776 : Inport  Description=|  Port=574  OutDataTypeStr=Nr2  PortDimensions=1
      | Battery Monitoring Sensor
      | <to>All</to>
      | <typ>Internal</typ>
      | <ReqID>131255</ReqID>
      | <InitValue>0</InitValue>
  CarCfg_CarCfg779 : Inport  Description=|  Port=575  OutDataTypeStr=Nr2  PortDimensions=1
      | FRONT SEATS LIE FLAT
      | <to>All</to>
      | <typ>Internal</typ>
      | <ReqID>267525</ReqID>
      | <InitValue>0</InitValue>
  CarCfg_CarCfg78 : Inport  Description=|  Port=576  OutDataTypeStr=Nr2  PortDimensions=1
      | HOMELINK FREQUENCY SETTING
      | <to>All</to>
      | <typ>Internal</typ>
      | <ReqID>NULL</ReqID>
      | <InitValue>0</InitValue>
  CarCfg_CarCfg787 : Inport  Description=|  Port=577  OutDataTypeStr=Nr2  PortDimensions=1
      | Mirror overload protection type
      | <to>All</to>
      | <typ>Internal</typ>
      | <ReqID>198565</ReqID>
      | <InitValue>0</InitValue>
  CarCfg_CarCfg789 : Inport  Description=|  Port=578  OutDataTypeStr=Nr2  PortDimensions=1
      | Wind Following Human Movement Function
      | <to>All</to>
      | <typ>Internal</typ>
      | <ReqID>/</ReqID>
      | <InitValue>0</InitValue>
  CarCfg_CarCfg79 : Inport  Description=|  Port=579  OutDataTypeStr=Nr2  PortDimensions=1
      | CAR SHARING FUNCTION
      | <to>All</to>
      | <typ>Internal</typ>